simulink library "Garbage"  (R2023a)
settings: Solver=ode45  StartTime=0.0  StopTime=10.0  RelTol=1e-3  EnableMultiTasking=on
undefined symbols (not defined in the file or in its folder): c, ending_column_index, ending_row_index, framebuffer_16x1, framebuffer_2x2, framebuffer_4x4, framebuffer_8x8, HEIGHT, hot, POS_X, POS_Y, starting_column_index, starting_row_index, WIDTH
libraries: Memory_Blocks_Lib x459, simulink x235, simulink_extras x132, dspmtrx3 x116, Bit_Multiplexers_Lib x67, Bit_Demultiplexers_Lib x49, Byte_Multiplexers_Lib x40, Miscellaneous x38, Computer_Library x17, Decoders_Lib x14, commutil2 x12, Arithmetic_Operations_Lib x8
(part 1 of 26)
settings Model: Creator=N  LastModifiedBy=Jozko Mrkvicka
settings SolverCC: AlgebraicLoopSolver=TrustRegion
settings DataIOCC: LimitDataPoints=on  SaveFormat=Array  ReturnWorkspaceOutputs=off
settings OptimizationCC: LifeSpan=inf
settings DebuggingCC: UnconnectedInputMsg=warning  UnconnectedOutputMsg=warning  UnconnectedLineMsg=warning  SFUnexpectedBacktrackingDiag=warning  SFNoUnconditionalDefaultTransitionDiag=warning  ArithmeticOperatorsInVariantConditions=warning
settings ModelReferenceCC: EnableRefExpFcnMdlSchedulingChecks=on
settings SFSimCC: SimUseLocalCustomCode=off  CustomCodeUndefinedFunction=UseInterfaceOnly
settings RTWCC: RTWUseLocalCustomCode=off
settings CodeAppCC: StateflowObjectComments=on
settings GRTTargetCC: TargetLangStandard=C89/C90 (ANSI)
settings ConfigComp: CovMetricSettings=dw  CovSaveCumulativeToWorkspaceVar=on  CovSaveSingleToWorkspaceVar=on  CovExternalEMLEnable=off

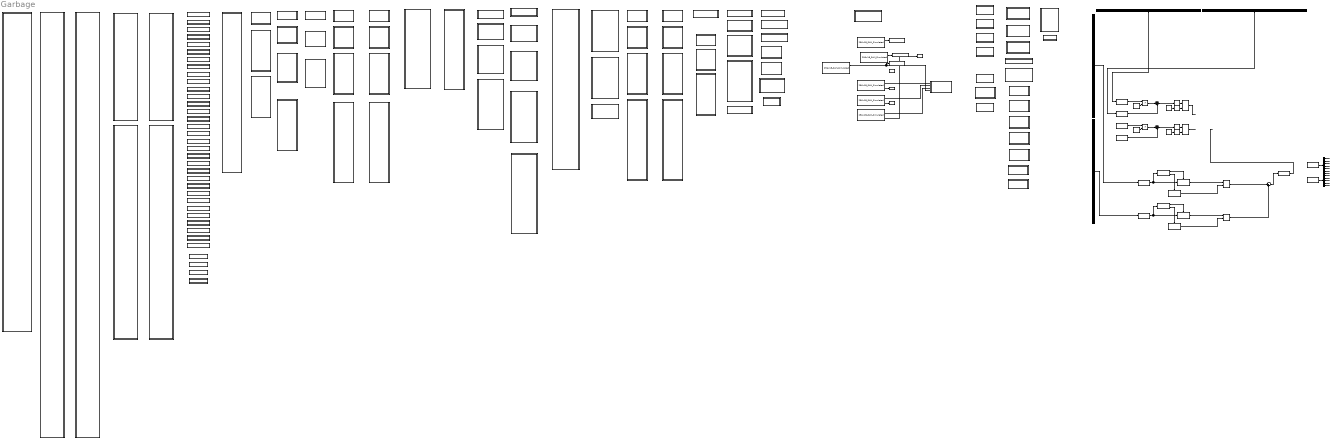
== system Garbage ==
  note: log2(n)
  note: Left shift
  note: log2(n)
  note: Left shift
  16x1B_RAM : Subsystem
  16x2B_RAM_Memory_Pokus_Merge : Subsystem
  256x1B_RAM : Subsystem
  256x1B_RAM_0_Simulated : Memory_Blocks_Lib/Memory_Blocks_Lib/256x1B_RAM_Simulated  SystemSampleTime=-1  SSRTWFcnClass=[]  VariantActivationTime=update diagram  Latency=0  AutoFrameSizeCalculation=off
      settings: LibrarySourceBlock=Memory_Blocks_Lib/Memory_Blocks_Lib/256x1B_RAM_Simulated
  256x1B_RAM_1_Simulated : Memory_Blocks_Lib/Memory_Blocks_Lib/256x1B_RAM_Simulated  SystemSampleTime=-1  SSRTWFcnClass=[]  VariantActivationTime=update diagram  Latency=0  AutoFrameSizeCalculation=off
      settings: LibrarySourceBlock=Memory_Blocks_Lib/Memory_Blocks_Lib/256x1B_RAM_Simulated
  256x1B_ROM : Subsystem
  256x1B_VideoRAM_0_Simulated : Memory_Blocks_Lib/Memory_Blocks_Lib/256x1B_RAM_Simulated  SystemSampleTime=-1  SSRTWFcnClass=[]  VariantActivationTime=update diagram  Latency=0  AutoFrameSizeCalculation=off
      settings: LibrarySourceBlock=Memory_Blocks_Lib/Memory_Blocks_Lib/256x1B_RAM_Simulated
  256x1B_VideoRAM_1_Simulated : Memory_Blocks_Lib/Memory_Blocks_Lib/256x1B_RAM_Simulated  SystemSampleTime=-1  SSRTWFcnClass=[]  VariantActivationTime=update diagram  Latency=0  AutoFrameSizeCalculation=off
      settings: LibrarySourceBlock=Memory_Blocks_Lib/Memory_Blocks_Lib/256x1B_RAM_Simulated
  256x1B_VideoRAM_2_Simulated : Memory_Blocks_Lib/Memory_Blocks_Lib/256x1B_RAM_Simulated  SystemSampleTime=-1  SSRTWFcnClass=[]  VariantActivationTime=update diagram  Latency=0  AutoFrameSizeCalculation=off
      settings: LibrarySourceBlock=Memory_Blocks_Lib/Memory_Blocks_Lib/256x1B_RAM_Simulated
  256x1B_VideoRAM_3_Simulated : Memory_Blocks_Lib/Memory_Blocks_Lib/256x1B_RAM_Simulated  SystemSampleTime=-1  SSRTWFcnClass=[]  VariantActivationTime=update diagram  Latency=0  AutoFrameSizeCalculation=off
      settings: LibrarySourceBlock=Memory_Blocks_Lib/Memory_Blocks_Lib/256x1B_RAM_Simulated
  32B_MPROM_Memory : Subsystem
  "32B_RAM_Memory " : Subsystem
  32B_VRAM : Subsystem
  "32B_VRAM " : Subsystem
  <0x0000..0x0FFF> : Subsystem
  <0x2000..0x23FF> : Subsystem
  <0xFFF6..0xFFF6> : Subsystem
  <0xFFFE..0xFFFE> : Subsystem
  Bit to Integer Converter{1,3..7} (6 alike) : simulink/Logic and Bit Operations/Bit to Integer Converter  nbits=32  bitOrder=MSB first  signedOutputValues=Unsigned  outDtype=double  outDtypeSigned=Inherit via internal rule
      settings: LibrarySourceBlock=|
      | commutil2/Bit to Integer
      | Converter
  Bit_Demultiplexer_1_to_256 : Subsystem
  Bit_Demux_16 : Subsystem
  Bit_Demux_4 : Subsystem
  Bit_Demux_8 : Subsystem
  Bit_Mux_16_Nand : Subsystem
  Bit_Mux_2_Nand : Subsystem
  Bit_Mux_4_Nand : Subsystem
  Bit_Mux_8_Nand : Subsystem
  Byte : Subsystem
  Byte_Demultiplexer_1_to_16 : Subsystem
  Byte_Enabled : Subsystem
  Byte_Multiplexer_16_to_1 : Subsystem
  Byte_Multiplexer_2_to_1 : Subsystem
  Byte_Multiplexer_4_to_1 : Subsystem
  Byte_Multiplexer_8_to_1 : Subsystem
  Constant1 : Constant  Value=2^32
  Constant2 : Constant  Value=2
  Constant3 : Constant  Value=2
  Constant4 : Constant  Value=2^32
  Counter_15bit : Subsystem
  Counter_8bit_Cfg : Subsystem
  Counter_8bit_Cfg__ : Subsystem
  Dec_16 : Subsystem  TreatAsAtomicUnit=on
  Dec_16_Enbl : Subsystem  TreatAsAtomicUnit=on
  Dec_16_Enbl_Invr : Subsystem  TreatAsAtomicUnit=on
  Dec_16_Invr : Subsystem  TreatAsAtomicUnit=on
  Dec_32 : Subsystem  TreatAsAtomicUnit=on
  Dec_32_Enbl : Subsystem  TreatAsAtomicUnit=on
  Dec_32_Enbl_Invr : Subsystem  TreatAsAtomicUnit=on
  Dec_32_Invr : Subsystem  TreatAsAtomicUnit=on
  Dec_4 : Subsystem  TreatAsAtomicUnit=on
  Dec_4_Enbl : Subsystem  TreatAsAtomicUnit=on
  Dec_4_Enbl_Invr : Subsystem  TreatAsAtomicUnit=on
  Dec_4_Invr : Subsystem  TreatAsAtomicUnit=on
  Dec_8 : Subsystem  TreatAsAtomicUnit=on
  Dec_8_Enbl : Subsystem  TreatAsAtomicUnit=on
  Dec_8_Enbl_Invr : Subsystem  TreatAsAtomicUnit=on
  Dec_8_Invr : Subsystem  TreatAsAtomicUnit=on
  Decoder_8_to_256_Enable : Subsystem  TreatAsAtomicUnit=on
  Delay{3..6} (4 alike) : Miscellaneous/Delay
  Demux2 : Demux  Outputs=6
  Demux3 : Demux  Outputs=6
  Display_16x1_Characters : Subsystem
  Display_2x2_Characters : Subsystem
  Display_4x4_Characters : Subsystem
  Display_8x8 : Subsystem
  Encoder_128_to_7 : Subsystem
  Graphic_Display_16x24 : Subsystem
  If : If  IfExpression=u1 ~= 0
  If1 : If  IfExpression=u1 ~= 0
  Integer to Bit Converter1 : simulink/Logic and Bit Operations/Integer to Bit Converter  nbits=6  signedInputValues=Unsigned  bitOrder=MSB first  outDtype=boolean
      settings: LibrarySourceBlock=|
      | commutil2/Integer to Bit
      | Converter
  Integer to Bit Converter2 : simulink/Logic and Bit Operations/Integer to Bit Converter  nbits=6  signedInputValues=Unsigned  bitOrder=MSB first  outDtype=boolean
      settings: LibrarySourceBlock=|
      | commutil2/Integer to Bit
      | Converter
  Integer to Bit Converter3 : simulink/Logic and Bit Operations/Integer to Bit Converter  nbits=6  signedInputValues=Unsigned  bitOrder=MSB first  outDtype=boolean
      settings: LibrarySourceBlock=|
      | commutil2/Integer to Bit
      | Converter
  Keyboard1 : Subsystem
  Log{1..4} (4 alike) : Math  Operator=log10
  "Log2 " : Subsystem  TreatAsAtomicUnit=on
  Log2 1 : Subsystem  TreatAsAtomicUnit=on
  Merge : Merge
  Merge1 : Merge
  MergeOutputs : Subsystem
  Mux{2..5} (4 alike) : Mux  Inputs=32
  NOT_USED_Bit_Subtractor : Subsystem
  NOT_USED_Byte_Demultiplexer_1_to_2 : Subsystem
  NOT_USED_Byte_Demultiplexer_1_to_4 : Subsystem
  NOT_USED_Byte_Demultiplexer_1_to_8 : Subsystem
  NOT_USED_Byte_Multiplexer_16_to_1_DirSel : Subsystem
  NOT_USED_Byte_Subtractor : Subsystem
  NOT_USED_Counter_2bit : Subsystem
  NOT_USED_Counter_3bit : Subsystem
  NOT_USED_Counter_4bit : Subsystem
  NOT_USED_Counter_4bit_Hours : Subsystem
  NOT_USED_Decoder_6_to_64 : Subsystem  TreatAsAtomicUnit=on
  NOT_USED_Decoder_6_to_64_Enable : Subsystem  TreatAsAtomicUnit=on
  NOT_USED_Decoder_7_to_128 : Subsystem  TreatAsAtomicUnit=on
  NOT_USED_Decoder_7_to_128_Enable : Subsystem  TreatAsAtomicUnit=on
  NOT_USED_Decoder_8_to_256 : Subsystem  TreatAsAtomicUnit=on
  NOT_USED_Text_Memory_16x1_Characters : Subsystem
  NOT_USED_Text_Memory_2x2_Characters : Subsystem
  NOT_USED_Text_Memory_4x4_Characters : Subsystem
  NOT_USED_Text_Memory_8x8_Characters : Subsystem
  Nibble_Mux_16 : Subsystem
  Nibble_Mux_2 : Subsystem
  Nibble_Mux_4 : Subsystem
  Nibble_Mux_8 : Subsystem
  Product1 : Product  Inputs=**
  Product2 : Product  Inputs=*/  OutDataTypeStr=uint8
  Product3 : Product  Inputs=*/  OutDataTypeStr=uint8
  Product4 : Product  Inputs=**
  Screen_40x25__ : Subsystem
  Selector_00 : Subsystem
  Selector_01 : Subsystem
  Selector_02 : Subsystem
  Selector_03 : Subsystem
  Selector_04 : Subsystem
  Selector_05 : Subsystem
  Selector_06 : Subsystem
  Selector_07 : Subsystem
  Selector_08 : Subsystem
  Selector_09 : Subsystem
  Selector_10 : Subsystem
  Selector_11 : Subsystem
  Selector_12 : Subsystem
  Selector_13 : Subsystem
  Selector_14 : Subsystem
  Selector_15 : Subsystem
  Selector_16 : Subsystem
  Selector_17 : Subsystem
  Selector_18 : Subsystem
  Selector_19 : Subsystem
  Selector_20 : Subsystem
  Selector_21 : Subsystem
  Selector_22 : Subsystem
  Selector_23 : Subsystem
  Selector_24 : Subsystem
  Selector_25 : Subsystem
  Selector_26 : Subsystem
  Selector_27 : Subsystem
  Selector_28 : Subsystem
  Selector_29 : Subsystem
  Selector_30 : Subsystem
  Selector_31 : Subsystem
  Stack_Pointer_Register : Subsystem
  Stack_write_violation_unfinished : Subsystem
  Sum1 : Sum  Inputs=|++
  Sum2 : Sum  Inputs=|++
  Sum3 : Sum  Inputs=|++  OutDataTypeStr=uint8
  Text_Display_1x16 : Subsystem
  To Workspace1 : ToWorkspace  VariableName=DataBus_512_767  SampleTime=-1
      settings: MaxDataPoints=1  Save2DSignal=2-D array (concatenate along first dimension)
  To Workspace11 : ToWorkspace  VariableName=DataBus_256_511  SampleTime=-1
      settings: MaxDataPoints=1  Save2DSignal=2-D array (concatenate along first dimension)
  To Workspace5 : ToWorkspace  VariableName=DataBus_768_1023  SampleTime=-1
      settings: MaxDataPoints=1  Save2DSignal=2-D array (concatenate along first dimension)
  Word : Subsystem
  Word_Enable_Action : Subsystem
  Word_Enabled : Subsystem
  Word_Enabled1 : Subsystem
  Word_Multiplexer_16_to_1 : Subsystem
  Word_Multiplexer_16_to_1_DirSel : Subsystem
  Word_Multiplexer_2_to_1 : Subsystem
  Word_Multiplexer_4_to_1 : Subsystem
  Word_Multiplexer_8_to_1 : Subsystem
  Zero : Subsystem  TreatAsAtomicUnit=on
  Zero1 : Subsystem  TreatAsAtomicUnit=on
  __1_to_256_Byte_Demultiplexer__ : Subsystem
  wires:
  256x1B_VideoRAM_2_Simulated/1 -> Screen_40x25__/2(Data_2(256x1B))
  256x1B_VideoRAM_2_Simulated/2 -> Delay5/1
  256x1B_RAM_1_Simulated/1 -> To Workspace1/1
  256x1B_VideoRAM_3_Simulated/2 -> Delay6/1
  256x1B_RAM_0_Simulated/1 -> To Workspace11/1
  256x1B_VideoRAM_3_Simulated/1 -> Screen_40x25__/1(Data_3(256x1B))
  256x1B_VideoRAM_1_Simulated/2 -> Delay4/1
  256x1B_VideoRAM_1_Simulated/1 -> Screen_40x25__/3(Data_1(256x1B))
  256x1B_VideoRAM_0_Simulated/1 -> To Workspace5/1, Screen_40x25__/4(Data_0(256x1B))
  Product3/1 -> ?
  Demux2/2 -> ?
  Demux2/5 -> ?
  Demux3/6 -> ?
  Product4/1 -> Sum1/1
  Bit to Integer Converter5/1 -> Sum1/2
  Zero/1(Out1) -> Merge/2
  Demux3/3 -> ?
  Log1/1 -> Product3/2
  Constant4/1 -> Product4/2
  Constant2/1 -> Log2/1
  Sum2/1 -> Log3/1
  Bit to Integer Converter3/1 -> Sum2/2
  Demux3/2 -> ?
  Constant1/1 -> Product1/2
  Log2/1 -> Product2/2
  Mux5/1 -> Bit to Integer Converter7/1
  Zero1/1(Out1) -> Merge1/2
  Integer to Bit Converter1/1 -> ?
  Demux2/4 -> ?
  Sum3/1 -> Integer to Bit Converter1/1
  Log2 1/1(Out1) -> Merge1/1
  If1/2 -> Zero1/Action
  Demux2/3 -> ?
  Bit to Integer Converter7/1 -> If/1, "Log2 "/1(In1)
  Merge/1 -> Sum3/2
  Bit to Integer Converter6/1 -> If1/1, Log2 1/1(In1)
  Merge1/1 -> Sum3/1
  Mux3/1 -> Bit to Integer Converter1/1
  Demux3/4 -> ?
  Demux3/1 -> ?
  Mux4/1 -> Bit to Integer Converter6/1
  Demux3/5 -> ?
  Constant3/1 -> Log1/1
  Sum1/1 -> Log4/1
  Product1/1 -> Sum2/1
  If1/1 -> Log2 1/Action
  Bit to Integer Converter4/1 -> Product4/1
  "Log2 "/1(Out1) -> Merge/1
  Mux2/1 -> Bit to Integer Converter3/1
  Log4/1 -> Product3/1
  Integer to Bit Converter3/1 -> Demux2/1
  If/1 -> "Log2 "/Action
  Integer to Bit Converter2/1 -> Demux3/1
  Log3/1 -> Product2/1
  If/2 -> Zero/Action
  Demux2/1 -> ?
  Bit to Integer Converter1/1 -> Product1/1
  Demux2/6 -> ?
  Product2/1 -> ?

== system Garbage/16x1B_RAM ==
  Addr(2B) : Inport
  Data(1B) : Inport  Port=2
  Write(1b) : Inport  Port=3
  CLK : Inport  Port=4  OutDataTypeStr=double
  4_to_16_Decoder : Decoders_Lib/Decoders_Lib/Decoder_4_to_16
  Bus Selector1 : BusSelector  OutputSignals=signal13,signal14,signal15,signal16
  Byte_00 : Memory_Blocks_Lib/Memory_Blocks_Lib/Byte  SystemSampleTime=-1  VariantActivationTime=update diagram  Latency=0  AutoFrameSizeCalculation=off
  Byte_01 : Memory_Blocks_Lib/Memory_Blocks_Lib/Byte  SystemSampleTime=-1  VariantActivationTime=update diagram  Latency=0  AutoFrameSizeCalculation=off
  Byte_02 : Memory_Blocks_Lib/Memory_Blocks_Lib/Byte  SystemSampleTime=-1  VariantActivationTime=update diagram  Latency=0  AutoFrameSizeCalculation=off
  Byte_03 : Memory_Blocks_Lib/Memory_Blocks_Lib/Byte  SystemSampleTime=-1  VariantActivationTime=update diagram  Latency=0  AutoFrameSizeCalculation=off
  Byte_04 : Memory_Blocks_Lib/Memory_Blocks_Lib/Byte  SystemSampleTime=-1  VariantActivationTime=update diagram  Latency=0  AutoFrameSizeCalculation=off
  Byte_05 : Memory_Blocks_Lib/Memory_Blocks_Lib/Byte  SystemSampleTime=-1  VariantActivationTime=update diagram  Latency=0  AutoFrameSizeCalculation=off
  Byte_06 : Memory_Blocks_Lib/Memory_Blocks_Lib/Byte  SystemSampleTime=-1  VariantActivationTime=update diagram  Latency=0  AutoFrameSizeCalculation=off
  Byte_07 : Memory_Blocks_Lib/Memory_Blocks_Lib/Byte  SystemSampleTime=-1  VariantActivationTime=update diagram  Latency=0  AutoFrameSizeCalculation=off
  Byte_08 : Memory_Blocks_Lib/Memory_Blocks_Lib/Byte  SystemSampleTime=-1  VariantActivationTime=update diagram  Latency=0  AutoFrameSizeCalculation=off
  Byte_09 : Memory_Blocks_Lib/Memory_Blocks_Lib/Byte  SystemSampleTime=-1  VariantActivationTime=update diagram  Latency=0  AutoFrameSizeCalculation=off
  Byte_{10..15} (6 alike) : Memory_Blocks_Lib/Memory_Blocks_Lib/Byte  SystemSampleTime=-1  VariantActivationTime=update diagram  Latency=0  AutoFrameSizeCalculation=off
  Merge : Merge  Inputs=16
  Vector Concatenate : Concatenate  NumInputs=16
  DataOut(1B) : Outport
  Video(16x1B) : Outport  Port=2  PortDimensions=16
  wires:
  Bus Selector1/4 -> 4_to_16_Decoder/4  "<signal16>"
  Bus Selector1/3 -> 4_to_16_Decoder/3  "<signal15>"
  Bus Selector1/2 -> 4_to_16_Decoder/2  "<signal14>"
  CLK/1 -> Byte_15/3, Byte_14/3, Byte_13/3, Byte_12/3, Byte_11/3, Byte_10/3, Byte_09/3, Byte_08/3, Byte_07/3, Byte_06/3, Byte_05/3, Byte_04/3, Byte_03/3, Byte_02/3, Byte_01/3, Byte_00/3
  Data(1B)/1 -> Byte_15/2, Byte_14/2, Byte_13/2, Byte_12/2, Byte_11/2, Byte_10/2, Byte_09/2, Byte_08/2, Byte_07/2, Byte_06/2, Byte_05/2, Byte_04/2, Byte_03/2, Byte_02/2, Byte_01/2, Byte_00/2
  Write(1b)/1 -> Byte_15/1, Byte_14/1, Byte_13/1, Byte_12/1, Byte_11/1, Byte_10/1, Byte_09/1, Byte_08/1, Byte_07/1, Byte_06/1, Byte_05/1, Byte_04/1, Byte_03/1, Byte_02/1, Byte_01/1, Byte_00/1
  4_to_16_Decoder/1 -> Byte_15/Enable
  Bus Selector1/1 -> 4_to_16_Decoder/1  "<signal13>"
  4_to_16_Decoder/2 -> Byte_14/Enable
  4_to_16_Decoder/3 -> Byte_13/Enable
  4_to_16_Decoder/4 -> Byte_12/Enable
  4_to_16_Decoder/5 -> Byte_11/Enable
  4_to_16_Decoder/6 -> Byte_10/Enable
  4_to_16_Decoder/7 -> Byte_09/Enable
  4_to_16_Decoder/8 -> Byte_08/Enable
  4_to_16_Decoder/9 -> Byte_07/Enable
  4_to_16_Decoder/10 -> Byte_06/Enable
  4_to_16_Decoder/11 -> Byte_05/Enable
  4_to_16_Decoder/12 -> Byte_04/Enable
  4_to_16_Decoder/13 -> Byte_03/Enable
  4_to_16_Decoder/14 -> Byte_02/Enable
  4_to_16_Decoder/15 -> Byte_01/Enable
  4_to_16_Decoder/16 -> Byte_00/Enable
  Byte_14/1 -> Merge/2
  Byte_12/1 -> Merge/4
  Byte_11/1 -> Merge/5
  Byte_10/1 -> Merge/6
  Byte_09/1 -> Merge/7
  Byte_08/1 -> Merge/8
  Byte_07/1 -> Merge/9
  Byte_06/1 -> Merge/10
  Byte_05/1 -> Merge/11
  Byte_04/1 -> Merge/12
  Byte_03/1 -> Merge/13
  Byte_02/1 -> Merge/14
  Byte_01/1 -> Merge/15
  Merge/1 -> DataOut(1B)/1
  Addr(2B)/1 -> Bus Selector1/1
  Byte_13/1 -> Merge/3
  Byte_15/1 -> Merge/1
  ? -> Vector Concatenate/16
  ? -> Vector Concatenate/15
  ? -> Vector Concatenate/14
  ? -> Vector Concatenate/13
  ? -> Vector Concatenate/12
  ? -> Vector Concatenate/11
  ? -> Vector Concatenate/10
  ? -> Vector Concatenate/9
  ? -> Vector Concatenate/8
  ? -> Vector Concatenate/7
  ? -> Vector Concatenate/6
  ? -> Vector Concatenate/5
  ? -> Vector Concatenate/4
  ? -> Vector Concatenate/3
  ? -> Vector Concatenate/2
  ? -> Vector Concatenate/1
  Byte_00/1 -> Merge/16
  Vector Concatenate/1 -> Video(16x1B)/1

== system Garbage/16x2B_RAM_Memory_Pokus_Merge ==
  Addr(2B) : Inport
  Data(2B) : Inport  Port=2
  Write(1b) : Inport  Port=3
  CLK : Inport  Port=4  OutDataTypeStr=double
  4_to_16_Decoder : Decoders_Lib/Decoders_Lib/Decoder_4_to_16
  Bit to Integer Converter{17..32} (16 alike) : simulink/Logic and Bit Operations/Bit to Integer Converter  Commented=on  nbits=16  bitOrder=MSB first  signedOutputValues=Unsigned  outDtype=uint16  outDtypeSigned=Inherit via internal rule
      settings: LibrarySourceBlock=|
      | commutil2/Bit to Integer
      | Converter
  Bus Selector1 : BusSelector  OutputSignals=signal13,signal14,signal15,signal16
  Merge : Merge  Inputs=16
  Vector Concatenate : Concatenate  NumInputs=16
  Word_Enabled_{0..15} (16 alike) : Memory_Blocks_Lib/Memory_Blocks_Lib/Word_Enabled  SystemSampleTime=-1  VariantActivationTime=update diagram  Latency=0  AutoFrameSizeCalculation=off
  DataOut(2B) : Outport
  Video(16x2B) : Outport  Port=2  PortDimensions=16
  wires:
  Bus Selector1/4 -> 4_to_16_Decoder/4  "<signal16>"
  Bus Selector1/3 -> 4_to_16_Decoder/3  "<signal15>"
  Bus Selector1/2 -> 4_to_16_Decoder/2  "<signal14>"
  CLK/1 -> Word_Enabled_13/3, Word_Enabled_12/3, Word_Enabled_11/3, Word_Enabled_10/3, Word_Enabled_9/3, Word_Enabled_8/3, Word_Enabled_7/3, Word_Enabled_6/3, Word_Enabled_5/3, Word_Enabled_4/3, Word_Enabled_3/3, Word_Enabled_2/3, Word_Enabled_1/3, Word_Enabled_0/3, Word_Enabled_14/3, Word_Enabled_15/3
  Data(2B)/1 -> Word_Enabled_13/2, Word_Enabled_12/2, Word_Enabled_11/2, Word_Enabled_10/2, Word_Enabled_9/2, Word_Enabled_8/2, Word_Enabled_7/2, Word_Enabled_6/2, Word_Enabled_5/2, Word_Enabled_4/2, Word_Enabled_3/2, Word_Enabled_2/2, Word_Enabled_1/2, Word_Enabled_0/2, Word_Enabled_14/2, Word_Enabled_15/2
  Write(1b)/1 -> Word_Enabled_14/1, Word_Enabled_13/1, Word_Enabled_12/1, Word_Enabled_11/1, Word_Enabled_10/1, Word_Enabled_9/1, Word_Enabled_8/1, Word_Enabled_7/1, Word_Enabled_6/1, Word_Enabled_5/1, Word_Enabled_4/1, Word_Enabled_3/1, Word_Enabled_2/1, Word_Enabled_1/1, Word_Enabled_0/1, Word_Enabled_15/1
  4_to_16_Decoder/1 -> Word_Enabled_15/Enable
  Bus Selector1/1 -> 4_to_16_Decoder/1  "<signal13>"
  4_to_16_Decoder/2 -> Word_Enabled_14/Enable
  4_to_16_Decoder/3 -> Word_Enabled_13/Enable
  4_to_16_Decoder/4 -> Word_Enabled_12/Enable
  4_to_16_Decoder/5 -> Word_Enabled_11/Enable
  4_to_16_Decoder/6 -> Word_Enabled_10/Enable
  4_to_16_Decoder/7 -> Word_Enabled_9/Enable
  4_to_16_Decoder/8 -> Word_Enabled_8/Enable
  4_to_16_Decoder/9 -> Word_Enabled_7/Enable
  4_to_16_Decoder/10 -> Word_Enabled_6/Enable
  4_to_16_Decoder/11 -> Word_Enabled_5/Enable
  4_to_16_Decoder/12 -> Word_Enabled_4/Enable
  4_to_16_Decoder/13 -> Word_Enabled_3/Enable
  4_to_16_Decoder/14 -> Word_Enabled_2/Enable
  4_to_16_Decoder/15 -> Word_Enabled_1/Enable
  4_to_16_Decoder/16 -> Word_Enabled_0/Enable
  Word_Enabled_14/1 -> Merge/2
  Word_Enabled_12/1 -> Merge/4
  Word_Enabled_11/1 -> Merge/5
  Word_Enabled_10/1 -> Merge/6
  Word_Enabled_9/1 -> Merge/7
  Word_Enabled_8/1 -> Merge/8
  Word_Enabled_7/1 -> Merge/9
  Word_Enabled_6/1 -> Merge/10
  Word_Enabled_5/1 -> Merge/11
  Word_Enabled_4/1 -> Merge/12
  Word_Enabled_3/1 -> Merge/13
  Word_Enabled_2/1 -> Merge/14
  Word_Enabled_1/1 -> Merge/15
  Merge/1 -> DataOut(2B)/1
  Addr(2B)/1 -> Bus Selector1/1
  Word_Enabled_13/1 -> Merge/3
  Word_Enabled_15/1 -> Merge/1
  ? -> Vector Concatenate/16
  ? -> Vector Concatenate/15
  ? -> Vector Concatenate/14
  ? -> Vector Concatenate/13
  ? -> Vector Concatenate/12
  ? -> Vector Concatenate/11
  ? -> Vector Concatenate/10
  ? -> Vector Concatenate/9
  ? -> Vector Concatenate/8
  ? -> Vector Concatenate/7
  ? -> Vector Concatenate/6
  ? -> Vector Concatenate/5
  ? -> Vector Concatenate/4
  ? -> Vector Concatenate/3
  ? -> Vector Concatenate/2
  ? -> Vector Concatenate/1
  Word_Enabled_0/1 -> Merge/16
  Vector Concatenate/1 -> Video(16x2B)/1
